simulink model "ofdm_txrx_supermimo_coded"  (Simulink 7.7)
settings: Solver=VariableStepDiscrete  SolverMode=SingleTasking  StartTime=0.0  StopTime=2*simOnly_simLength  RelTol=1e-3
PostLoadFcn:
  | ofdm_rx_supermimo_init
InitFcn:
  | ofdm_rx_supermimo_init
StartFcn:
  | ofdm_rx_supermimo_init
values from neighbouring files (symbols this model uses):
  ActionID_AFTransmit = 31    [ofdm_tx_supermimo_init.m]
  ActionID_Disabled = 0    [ofdm_tx_supermimo_init.m]
  ActionID_SetFlagA = 62    [ofdm_tx_supermimo_init.m]
  ActionID_SetFlagB = 61    [ofdm_tx_supermimo_init.m]
  BER_RAM_init_size = 2048    [ofdm_tx_supermimo_init.m]
  channel_h = [1 0; 1 0] * 0.3    [ofdm_tx_supermimo_init.m]
  CPLength = 16    [ofdm_tx_supermimo_init.m]
  longCorr_coef_i = [-4*real(LTS) 0]    [ofdm_rx_supermimo_init.m]
  longCorr_coef_q = [4*imag(LTS) 0]    [ofdm_rx_supermimo_init.m]
  max_CP_length = 16    [ofdm_tx_supermimo_init.m]
  max_num_baseRateSymbols = 31    [ofdm_tx_supermimo_init.m]
  max_num_subcarriers = 64    [ofdm_tx_supermimo_init.m]
  max_num_trainingSymbols = 15    [ofdm_tx_supermimo_init.m]
  max_numBytes = 16384    [ofdm_tx_supermimo_init.m]
  max_OFDM_symbols = 1023    [ofdm_tx_supermimo_init.m]
  modConstellation_qam16 = .75*[1 3 -1 -3]./3    [ofdm_tx_supermimo_init.m]
  modConstellation_qam64 = 0.875*[3 1 5 7 -3 -1 -5 -7]./7    [ofdm_tx_supermimo_init.m]
  modConstellation_qpsk = [1 -1]./sqrt(2)    [ofdm_tx_supermimo_init.m]
  numBaseRateSymbols = numBaseRateSymbols *2    [ofdm_tx_supermimo_init.m]
  numSubcarriers = 64    [ofdm_tx_supermimo_init.m]
  numTrainingSymbols = 2    [ofdm_tx_supermimo_init.m]
  pktTiming_count_payloadStart = pktTiming_count_lsStart + pktTiming_ls_num*pktTiming_ls_length    [ofdm_rx_supermimo_init.m]
  preamble = preamble_scale*[shortsyms_10 longsyms_2]    [ofdm_tx_supermimo_init.m]
  preamble_ROM = preamble_I_ROM + preamble_Q_ROM*2^16    [ofdm_tx_supermimo_init.m]
  RAM_init_size = max_numBytes    (= 16384)    [ofdm_tx_supermimo_init.m]
  RxDataScrambling_Seq = [40 198 78 63 82 173 102 245 48 111 172 115 147 230 216 93 72 65 62 2 205 242 122 90 128 83 105 97 73 10 5 252]    [ofdm_tx_supermimo_init.m]
  shortSymbol_time = shortSymbol_time(1:16).'    [ofdm_tx_supermimo_init.m]
  simOnly_numSamples = length(preamble)+( (numSubcarriers+CPLength)*(numTrainingSymbols + numBaseRateSymbols + numFullRateSymbols/2) )    [ofdm_tx_supermimo_init.m]
  train = [train train]    [ofdm_tx_supermimo_init.m]
  TxDataScrambling_Seq = [40 198 78 63 82 173 102 245 48 111 172 115 147 230 216 93 72 65 62 2 205 242 122 90 128 83 105 97 73 10 5 252]    [ofdm_tx_supermimo_init.m]
undefined symbols (not defined in the file or in its folder): AntA, AntB, BaseRate, Down, gcbh, numBuffs, numBytes, Up, xlBlockLoadCallback, xlGetNormalizedPeriod, xlipgetpartsetting
libraries: xbsIndex_r4 x5430
(part 13 of 49)

== system ofdm_txrx_supermimo_coded/OFDM Rx MIMO/Long Corr HP/C4 ==
  x : Inport
  AddSub : xbsIndex_r4/AddSub  mode=Addition  use_carryin=off  use_carryout=off  en=off  latency=0  precision=Full  arith_type=Unsigned  n_bits=8  bin_pt=2  quantization=Truncate  overflow=Wrap  dbl_ovrd=off  use_behavioral_HDL=on  hw_selection=Fabric  pipelined=off  use_rpm=off  block_type=addsub
      settings: xl_area=[3 0 0 4 0 0 0]  block_version=VER_STRING_GOES_HERE
  AddSub{1..14} (14 alike) : xbsIndex_r4/AddSub  mode=Addition  use_carryin=off  use_carryout=off  en=off  latency=1  precision=Full  arith_type=Unsigned  n_bits=8  bin_pt=2  quantization=Truncate  overflow=Wrap  dbl_ovrd=off  use_behavioral_HDL=on  hw_selection=Fabric  pipelined=off  use_rpm=off  block_type=addsub
      AddSub1 settings: xl_area=[3 5 0 4 0 0 0]  block_version=VER_STRING_GOES_HERE
      AddSub10 settings: xl_area=[3 5 0 4 0 0 0]  block_version=VER_STRING_GOES_HERE
      AddSub11 settings: xl_area=[3 5 0 4 0 0 0]  block_version=VER_STRING_GOES_HERE
      AddSub12 settings: xl_area=[3 5 0 4 0 0 0]  block_version=VER_STRING_GOES_HERE
      AddSub13 settings: xl_area=[3 6 0 5 0 0 0]  block_version=VER_STRING_GOES_HERE
      AddSub14 settings: xl_area=[3 6 0 5 0 0 0]  block_version=VER_STRING_GOES_HERE
      AddSub2 settings: xl_area=[3 6 0 5 0 0 0]  block_version=VER_STRING_GOES_HERE
      AddSub3 settings: xl_area=[3 5 0 4 0 0 0]  block_version=VER_STRING_GOES_HERE
      AddSub4 settings: xl_area=[4 7 0 6 0 0 0]  block_version=VER_STRING_GOES_HERE
      AddSub5 settings: xl_area=[3 5 0 4 0 0 0]  block_version=VER_STRING_GOES_HERE
      AddSub6 settings: xl_area=[3 6 0 5 0 0 0]  block_version=VER_STRING_GOES_HERE
      AddSub7 settings: xl_area=[3 5 0 4 0 0 0]  block_version=VER_STRING_GOES_HERE
      AddSub8 settings: xl_area=[4 8 0 7 0 0 0]  block_version=VER_STRING_GOES_HERE
      AddSub9 settings: xl_area=[4 7 0 6 0 0 0]  block_version=VER_STRING_GOES_HERE
  C1 : Subsystem  mask{coef2=h(1:N)}
      mask prompts: coef2 "Coefficients"
  C10 : Subsystem  mask{coef2=h(9*N+1:10*N)}
      mask prompts: coef2 "Coefficients"
  C11 : Subsystem  mask{coef2=h(10*N+1:11*N)}
      mask prompts: coef2 "Coefficients"
  C12 : Subsystem  mask{coef2=h(11*N+1:12*N)}
      mask prompts: coef2 "Coefficients"
  C13 : Subsystem  mask{coef2=h(12*N+1:13*N)}
      mask prompts: coef2 "Coefficients"
  C14 : Subsystem  mask{coef2=h(13*N+1:14*N)}
      mask prompts: coef2 "Coefficients"
  C15 : Subsystem  mask{coef2=h(14*N+1:15*N)}
      mask prompts: coef2 "Coefficients"
  C16 : Subsystem  mask{coef2=h(15*N+1:16*N)}
      mask prompts: coef2 "Coefficients"
  C2 : Subsystem  mask{coef2=h(N+1:2*N)}
      mask prompts: coef2 "Coefficients"
  C3 : Subsystem  mask{coef2=h(2*N+1:3*N)}
      mask prompts: coef2 "Coefficients"
  C4 : Subsystem  mask{coef2=h(3*N+1:4*N)}
      mask prompts: coef2 "Coefficients"
  C5 : Subsystem  mask{coef2=h(4*N+1:5*N)}
      mask prompts: coef2 "Coefficients"
  C6 : Subsystem  mask{coef2=h(5*N+1:6*N)}
      mask prompts: coef2 "Coefficients"
  C7 : Subsystem  mask{coef2=h(6*N+1:7*N)}
      mask prompts: coef2 "Coefficients"
  C8 : Subsystem  mask{coef2=h(7*N+1:8*N)}
      mask prompts: coef2 "Coefficients"
  C9 : Subsystem  mask{coef2=h(8*N+1:9*N)}
      mask prompts: coef2 "Coefficients"
  Delay{1,3..4,6,8,12..13,15} (8 alike) : xbsIndex_r4/Delay  rst=off  infoeditControl=Selection of Reset will increase slice count due to use of real FFs and instead of SRLs  en=off  latency=N-1  dbl_ovrd=off  reg_retiming=off  block_type=delay
      settings: infoedit=Hardware notes: A delay line is a chain, each link of which is an SRL16 followed by a flip-flop. If register retiming is enabled, the delay line is a chain of flip-flops.  xl_area=[2 4 0 4 0 0 0]  block_version=VER_STRING_GOES_HERE
  Delay10 : xbsIndex_r4/Delay  rst=off  infoeditControl=Selection of Reset will increase slice count due to use of real FFs and instead of SRLs  en=off  latency=((N-1)*12)-1  dbl_ovrd=off  reg_retiming=off  block_type=delay
      settings: infoedit=Hardware notes: A delay line is a chain, each link of which is an SRL16 followed by a flip-flop. If register retiming is enabled, the delay line is a chain of flip-flops.  xl_area=[9 15 0 15 0 0 0]  block_version=VER_STRING_GOES_HERE
  Delay11 : xbsIndex_r4/Delay  rst=off  infoeditControl=Selection of Reset will increase slice count due to use of real FFs and instead of SRLs  en=off  latency=((N-1)*14)-1  dbl_ovrd=off  reg_retiming=off  block_type=delay
      settings: infoedit=Hardware notes: A delay line is a chain, each link of which is an SRL16 followed by a flip-flop. If register retiming is enabled, the delay line is a chain of flip-flops.  xl_area=[9 15 0 15 0 0 0]  block_version=VER_STRING_GOES_HERE
  Delay14 : xbsIndex_r4/Delay  rst=off  infoeditControl=Selection of Reset will increase slice count due to use of real FFs and instead of SRLs  en=off  latency=((N-1)*2)-1  dbl_ovrd=off  reg_retiming=off  block_type=delay
      settings: infoedit=Hardware notes: A delay line is a chain, each link of which is an SRL16 followed by a flip-flop. If register retiming is enabled, the delay line is a chain of flip-flops.  xl_area=[3 5 0 5 0 0 0]  block_version=VER_STRING_GOES_HERE
  Delay2 : xbsIndex_r4/Delay  rst=off  infoeditControl=Selection of Reset will increase slice count due to use of real FFs and instead of SRLs  en=off  latency=((N-1)*4)-1  dbl_ovrd=off  reg_retiming=off  block_type=delay
      settings: infoedit=Hardware notes: A delay line is a chain, each link of which is an SRL16 followed by a flip-flop. If register retiming is enabled, the delay line is a chain of flip-flops.  xl_area=[3 5 0 5 0 0 0]  block_version=VER_STRING_GOES_HERE
  Delay5 : xbsIndex_r4/Delay  rst=off  infoeditControl=Selection of Reset will increase slice count due to use of real FFs and instead of SRLs  en=off  latency=((N-1)*6)-1  dbl_ovrd=off  reg_retiming=off  block_type=delay
      settings: infoedit=Hardware notes: A delay line is a chain, each link of which is an SRL16 followed by a flip-flop. If register retiming is enabled, the delay line is a chain of flip-flops.  xl_area=[6 10 0 10 0 0 0]  block_version=VER_STRING_GOES_HERE
  Delay7 : xbsIndex_r4/Delay  rst=off  infoeditControl=Selection of Reset will increase slice count due to use of real FFs and instead of SRLs  en=off  latency=((N-1)*8)-1  dbl_ovrd=off  reg_retiming=off  block_type=delay
      settings: infoedit=Hardware notes: A delay line is a chain, each link of which is an SRL16 followed by a flip-flop. If register retiming is enabled, the delay line is a chain of flip-flops.  xl_area=[6 10 0 10 0 0 0]  block_version=VER_STRING_GOES_HERE
  Delay9 : xbsIndex_r4/Delay  rst=off  infoeditControl=Selection of Reset will increase slice count due to use of real FFs and instead of SRLs  en=off  latency=((N-1)*10)-1  dbl_ovrd=off  reg_retiming=off  block_type=delay
      settings: infoedit=Hardware notes: A delay line is a chain, each link of which is an SRL16 followed by a flip-flop. If register retiming is enabled, the delay line is a chain of flip-flops.  xl_area=[9 15 0 15 0 0 0]  block_version=VER_STRING_GOES_HERE
  FSM : Subsystem
  Gateway Out6 : xbsIndex_r4/Gateway Out  inherit_from_input=off  hdl_port=off  timing_constraint=None  locs_specified=off  LOCs={}  UseAsDAC=off  DACChannel='1'  block_type=gatewayout
      settings: infoedit="Gateway out block.  Converts Xilinx fixed point inputs into ouputs of type Simulink integer, double, or fixed point.<P><P>Hardware notes:  In hardware these blocks become top level output ports or are discarded, depending on how they are configured."  xl_area=[0 0 0 0 0 0 0]  block_version=VER_STRING_GOES_HERE
  Gateway Out7 : xbsIndex_r4/Gateway Out  inherit_from_input=off  hdl_port=off  timing_constraint=None  locs_specified=off  LOCs={}  UseAsDAC=off  DACChannel='1'  block_type=gatewayout
      settings: infoedit="Gateway out block.  Converts Xilinx fixed point inputs into ouputs of type Simulink integer, double, or fixed point.<P><P>Hardware notes:  In hardware these blocks become top level output ports or are discarded, depending on how they are configured."  xl_area=[0 0 0 0 0 0 0]  block_version=VER_STRING_GOES_HERE
  Gateway Out8 : xbsIndex_r4/Gateway Out  inherit_from_input=off  hdl_port=off  timing_constraint=None  locs_specified=off  LOCs={}  UseAsDAC=off  DACChannel='1'  block_type=gatewayout
      settings: infoedit="Gateway out block.  Converts Xilinx fixed point inputs into ouputs of type Simulink integer, double, or fixed point.<P><P>Hardware notes:  In hardware these blocks become top level output ports or are discarded, depending on how they are configured."  xl_area=[0 0 0 0 0 0 0]  block_version=VER_STRING_GOES_HERE
  Long Corr Scope2 : Scope  NumInputPorts=3
      settings: YMin=-0.35~-0.175~0  YMax=0.35~0.225~1300  SaveName=ScopeData8
  y : Outport
  wires:
  AddSub9/1 -> Gateway Out8/1, AddSub8/2
  AddSub6/1 -> AddSub9/1, Gateway Out7/1
  C13/1(yn) -> AddSub10/1
  AddSub8/1 -> y/1
  Delay9/1 -> AddSub6/2
  Delay7/1 -> AddSub6/1
  C12/1(yn) -> Delay8/1
  AddSub7/1 -> Delay9/1
  Delay8/1 -> AddSub7/2
  C11/1(yn) -> AddSub7/1
  AddSub4/1 -> AddSub8/1
  AddSub2/1 -> AddSub4/2
  AddSub13/1 -> AddSub4/1
  Delay5/1 -> AddSub2/2
  Delay2/1 -> AddSub2/1
  AddSub/1 -> AddSub13/1
  Delay14/1 -> AddSub13/2
  C8/2(xnz) -> [xnz]
  C10/1(yn) -> Delay6/1
  AddSub5/1 -> Delay7/1
  Delay6/1 -> AddSub5/2
  C9/1(yn) -> AddSub5/1
  C8/1(yn) -> Delay4/1
  C7/1(yn) -> AddSub3/1
  AddSub3/1 -> Delay5/1
  Delay4/1 -> AddSub3/2
  C7/2(xnz) -> C8/1(xn)
  C6/1(yn) -> Delay1/1
  AddSub1/1 -> Delay2/1
  Delay1/1 -> AddSub1/2
  C5/1(yn) -> AddSub1/1
  C1/1(yn) -> AddSub/1
  Delay3/1 -> AddSub/2
  AddSub12/1 -> Delay14/1
  Delay13/1 -> AddSub12/2
  C12/2(xnz) -> C13/1(xn)
  C11/2(xnz) -> C12/1(xn)
  C10/2(xnz) -> C11/1(xn)
  C9/2(xnz) -> C10/1(xn)
  [xnz] -> C9/1(xn)
  C6/2(xnz) -> C7/1(xn)
  C5/2(xnz) -> C6/1(xn)
  C4/1(yn) -> Delay13/1
  C4/2(xnz) -> C5/1(xn)
  C3/1(yn) -> AddSub12/1
  C3/2(xnz) -> C4/1(xn)
  C2/2(xnz) -> C3/1(xn)
  FSM/3(load) -> C12/4(LD), C13/4(LD), C14/4(LD), C16/4(LD), C15/4(LD), C11/4(LD), C10/4(LD), C9/4(LD), C1/4(LD), C8/4(LD), C7/4(LD), C6/4(LD), C5/4(LD), C4/4(LD), C3/4(LD), C2/4(LD)
  FSM/2(Coef Addr) -> C12/3(CAddr), C13/3(CAddr), C14/3(CAddr), C16/3(CAddr), C15/3(CAddr), C11/3(CAddr), C10/3(CAddr), C9/3(CAddr), C1/3(CAddr), C8/3(CAddr), C7/3(CAddr), C6/3(CAddr), C5/3(CAddr), C4/3(CAddr), C3/3(CAddr), C2/3(CAddr)
  FSM/1(Data Addr) -> C12/2(DAddr), C13/2(DAddr), C14/2(DAddr), C16/2(DAddr), C15/2(DAddr), C11/2(DAddr), C10/2(DAddr), C9/2(DAddr), C1/2(DAddr), C8/2(DAddr), C7/2(DAddr), C6/2(DAddr), C5/2(DAddr), C4/2(DAddr), C3/2(DAddr), C2/2(DAddr)
  C1/2(xnz) -> C2/1(xn)
  C2/1(yn) -> Delay3/1
  x/1 -> C1/1(xn)
  C13/2(xnz) -> C14/1(xn)
  C14/2(xnz) -> C15/1(xn)
  C15/2(xnz) -> C16/1(xn)
  C15/1(yn) -> AddSub11/1
  C14/1(yn) -> Delay12/1
  C16/1(yn) -> Delay15/1
  Delay12/1 -> AddSub10/2
  Delay15/1 -> AddSub11/2
  AddSub10/1 -> Delay10/1
  AddSub11/1 -> Delay11/1
  Delay10/1 -> AddSub14/1
  Delay11/1 -> AddSub14/2
  AddSub14/1 -> AddSub9/2, Gateway Out6/1
  Gateway Out6/1 -> Long Corr Scope2/1
  Gateway Out7/1 -> Long Corr Scope2/2
  Gateway Out8/1 -> Long Corr Scope2/3

== system ofdm_txrx_supermimo_coded/OFDM Rx MIMO/Packet_Detection/Auto-Correlation Pkt Det Embedded MULT (for V2P/V6 export) ==
  note: Latency = 1
  note: Latency = 1
  AntA I : Inport
  AntA Q : Inport  Port=2
  PktDet Reset : Inport  Port=3
  A*conj(B) 4xCLK : Subsystem
  Coarse Pkt Det : Scope  NumInputPorts=7
      settings: TimeRange=2500  YMin=-1~-1~0.4~-0.09~12.75~-0.55~4  YMax=1~1~1.3~0.05~13.1~-0.05~4.275  SaveName=ScopeData28
  Coarse Pkt Det2 : Scope  NumInputPorts=7
      settings: TimeRange=2500  YMin=0~0~0~0~0~-1~0.95  YMax=0.3~0.08~0.8~0.7~0.45~1~1.05
  Convert1 : xbsIndex_r4/Convert  gui_display_data_type=Fixed-point  arith_type="Signed  (2's comp)"  n_bits=12  bin_pt=11  float_type=Single  exp_bits=8  fraction_bits=24  quantization=Truncate  overflow=Wrap  en=off  latency=0  dbl_ovrd=off  pipeline=off  block_type=convert
      settings: block_version=10.1.3
  Convert7 : xbsIndex_r4/Convert  gui_display_data_type=Fixed-point  arith_type="Signed  (2's comp)"  n_bits=12  bin_pt=11  float_type=Single  exp_bits=8  fraction_bits=24  quantization=Truncate  overflow=Wrap  en=off  latency=0  dbl_ovrd=off  pipeline=off  block_type=convert
      settings: block_version=10.1.3
  Delay : xbsIndex_r4/Delay  rst=off  infoeditControl=Selection of Reset will increase slice count due to use of real FFs and instead of SRLs  en=off  latency=16  dbl_ovrd=off  reg_retiming=off  block_type=delay
      settings: infoedit=Hardware notes: A delay line is a chain, each link of which is an SRL16 followed by a flip-flop. If register retiming is enabled, the delay line is a chain of flip-flops.  xl_area=[7 14 0 14 0 0 0]  block_version=VER_STRING_GOES_HERE
  Delay1 : xbsIndex_r4/Delay  rst=off  infoeditControl=Selection of Reset will increase slice count due to use of real FFs and instead of SRLs  en=off  latency=16  dbl_ovrd=off  reg_retiming=off  block_type=delay
      settings: infoedit=Hardware notes: A delay line is a chain, each link of which is an SRL16 followed by a flip-flop. If register retiming is enabled, the delay line is a chain of flip-flops.  xl_area=[7 14 0 14 0 0 0]  block_version=VER_STRING_GOES_HERE
  Detection Decision : Subsystem
  Display{,1..3} (4 alike) : Display
  Logical : xbsIndex_r4/Logical  logical_function=AND  inputs=2  en=off  latency=0  precision=Full  arith_type=Unsigned  n_bits=8  bin_pt=2  align_bp=on  dbl_ovrd=off  block_type=logical
      settings: xl_area=[1 0 0 1 0 0 0]  block_version=VER_STRING_GOES_HERE
  Magnitude Squared : Subsystem
  Magnitude Squared1 : Subsystem
  Mult1 : xbsIndex_r4/Mult  precision=User Defined  arith_type=Unsigned  n_bits=30  bin_pt=20  quantization=Truncate  overflow=Flag as error  en=off  latency=1  dbl_ovrd=off  use_behavioral_HDL=off  opt=Speed  use_embedded=on  optimum_pipeline=off  pipeline=on  use_rpm=off  placement_style=Rectangular shape  block_type=mult
      settings: xl_area=[63 105 0 106 0 4 0]  block_version=VER_STRING_GOES_HERE
  Mult2 : xbsIndex_r4/Mult  precision=User Defined  arith_type=Unsigned  n_bits=30  bin_pt=20  quantization=Truncate  overflow=Saturate  en=off  latency=1  dbl_ovrd=off  use_behavioral_HDL=off  opt=Speed  use_embedded=on  optimum_pipeline=off  pipeline=on  use_rpm=off  placement_style=Rectangular shape  block_type=mult
      settings: xl_area=[63 105 0 106 0 4 0]  block_version=VER_STRING_GOES_HERE
  Reinterpret : xbsIndex_r4/Reinterpret  force_arith_type=on  arith_type=Unsigned  force_bin_pt=on  bin_pt=0  block_type=reinterpret
  Reinterpret1 : xbsIndex_r4/Reinterpret  force_arith_type=on  arith_type=Unsigned  force_bin_pt=on  bin_pt=0  block_type=reinterpret
  Relational : xbsIndex_r4/Relational  mode=a>b  en=off  latency=1  dbl_ovrd=off  block_type=relational
      settings: xl_area=[11 1 0 22 0 0 0]  block_version=VER_STRING_GOES_HERE
  Relational1 : xbsIndex_r4/Relational  mode=a>b  en=off  latency=1  dbl_ovrd=off  block_type=relational
      settings: xl_area=[11 1 0 22 0 0 0]  block_version=VER_STRING_GOES_HERE
  Sliding Window : Subsystem
  Sliding Window1 : Subsystem
  Sliding Window2 : Subsystem
  done{1..18} (18 alike) : xbsIndex_r4/Gateway Out  inherit_from_input=off  hdl_port=off  timing_constraint=None  locs_specified=off  LOCs={}  UseAsDAC=off  DACChannel='1'  block_type=gatewayout
      settings: infoedit="Gateway out block.  Converts Xilinx fixed point inputs into ouputs of type Simulink integer, double, or fixed point.<P><P>Hardware notes:  In hardware these blocks become top level output ports or are discarded, depending on how they are configured."  xl_area=[0 0 0 0 1 0 0]  block_version=VER_STRING_GOES_HERE
  PktDet : Outport
  wires:
  Logical/1 -> Detection Decision/1(Det)
  Sliding Window2/1(Out) -> done10/1, Mult2/2, Mult2/1
  [pktDetCorr_minPower] -> Relational1/2, done15/1, Reinterpret1/1
  done13/1 -> Coarse Pkt Det2/6
  done12/1 -> Coarse Pkt Det2/5
  Mult2/1 -> Mult1/1, done11/1, Relational1/1
  [pktDetCorr_ratioThresh] -> Mult1/2, done14/1, Reinterpret/1
  done11/1 -> Coarse Pkt Det2/4
  done10/1 -> Coarse Pkt Det2/3
  done9/1 -> Coarse Pkt Det2/2
  done8/1 -> Coarse Pkt Det2/1
  Mult1/1 -> done12/1, Relational/2
  Magnitude Squared1/1(Mag) -> done9/1, Sliding Window2/1(In)
  done7/1 -> Coarse Pkt Det/7
  done3/1 -> Coarse Pkt Det/4
  Convert1/1 -> Magnitude Squared1/2(Q), done2/1, Delay1/1, A*conj(B) 4xCLK/3(Im[A])
  Convert7/1 -> Magnitude Squared1/1(I), done4/1, Delay/1, A*conj(B) 4xCLK/1(Re[A])
  done2/1 -> Coarse Pkt Det/2
  AntA Q/1 -> Convert1/1
  AntA I/1 -> Convert7/1
  Delay/1 -> A*conj(B) 4xCLK/2(Re[B])
  Delay1/1 -> A*conj(B) 4xCLK/4(Im[B])
  A*conj(B) 4xCLK/1(Re) -> done5/1, Sliding Window/1(In)
  A*conj(B) 4xCLK/2(Im1) -> done3/1, Sliding Window1/1(In)
  Sliding Window/1(Out) -> done6/1, Magnitude Squared/1(I)
  Sliding Window1/1(Out) -> done1/1, Magnitude Squared/2(Q)
  Magnitude Squared/1(Mag) -> Relational/1, done8/1, done7/1
  Relational/1 -> done13/1, Logical/1
  Relational1/1 -> Logical/2, done16/1
  done1/1 -> Coarse Pkt Det/6
  done4/1 -> Coarse Pkt Det/1
  done5/1 -> Coarse Pkt Det/3
  done6/1 -> Coarse Pkt Det/5
  done14/1 -> Display/1
  done15/1 -> Display1/1
  done16/1 -> Coarse Pkt Det2/7
  Reinterpret/1 -> done17/1
  done17/1 -> Display2/1
  Reinterpret1/1 -> done18/1
  done18/1 -> Display3/1
  PktDet Reset/1 -> Detection Decision/2(Reset)
  Detection Decision/1(Pkt Det) -> PktDet/1

== system ofdm_txrx_supermimo_coded/OFDM Rx MIMO/Packet_Detection/Ext PktDet ==
  Constant2 : Constant  Value=0
  Convert5 : xbsIndex_r4/Convert  gui_display_data_type=Fixed-point  arith_type=Boolean  n_bits=1  bin_pt=0  float_type=Single  exp_bits=8  fraction_bits=24  quantization=Truncate  overflow=Flag as error  en=off  latency=0  dbl_ovrd=off  pipeline=off  block_type=convert
      settings: block_version=10.1.3
  Down Sample1 : xbsIndex_r4/Down Sample  sample_ratio=4  sample_phase="Last Value of Frame  (most efficient)"  en=off  latency=1  dbl_ovrd=off  block_type=dsamp
      settings: block_version=VER_STRING_GOES_HERE
  Ext_PktDet : xbsIndex_r4/Gateway In  gui_display_data_type=Fixed-point  arith_type=Unsigned  n_bits=1  bin_pt=0  preci_type=Single  exp_width=8  frac_width=24  quantization="Round  (unbiased: +/- Inf)"  overflow=Saturate  period=1  dbl_ovrd=off  timing_constraint=None  locs_specified=off  LOCs={}  inherit_from_input=off  UseAsADC=off  ADCChannel='1'  hdl_port=on  block_type=gatewayin
      settings: infoedit="Gateway in block.  Converts inputs of type Simulink integer, double and fixed point to  Xilinx fixed point type.<P><P>Hardware notes:  In hardware these blocks become top level input ports."  block_version=VER_STRING_GOES_HERE
  Logical2 : xbsIndex_r4/Logical  logical_function=AND  inputs=2  en=off  latency=0  precision=Full  arith_type=Unsigned  n_bits=16  bin_pt=0  align_bp=on  dbl_ovrd=off  block_type=logical
      settings: block_version=8.2
  Det : Outport
  wires:
  Ext_PktDet/1 -> Down Sample1/1
  Constant2/1 -> Ext_PktDet/1
  [regPktDet_EnableExtDet] -> Logical2/2
  Logical2/1 -> Det/1
  Convert5/1 -> Logical2/1
  Down Sample1/1 -> Convert5/1

== system ofdm_txrx_supermimo_coded/OFDM Rx MIMO/Packet_Detection/Posedge ==
  D : Inport
  Delay : xbsIndex_r4/Delay  rst=off  infoeditControl=Selection of Reset will increase slice count due to use of real FFs and instead of SRLs  en=off  latency=1  dbl_ovrd=off  reg_retiming=off  block_type=delay
      settings: infoedit=Hardware notes: A delay line is a chain, each link of which is an SRL16 followed by a flip-flop. If register retiming is enabled, the delay line is a chain of flip-flops.  xl_area=[1 1 0 0 0 0 0]  block_version=VER_STRING_GOES_HERE
  Inverter : xbsIndex_r4/Inverter  en=off  latency=0  dbl_ovrd=off  block_type=inv
      settings: xl_area=[0 0 0 0 0 0 0]  block_version=VER_STRING_GOES_HERE
  Logical : xbsIndex_r4/Logical  logical_function=AND  inputs=2  en=off  latency=0  precision=Full  arith_type=Unsigned  n_bits=8  bin_pt=2  align_bp=on  dbl_ovrd=off  block_type=logical
      settings: xl_area=[1 0 0 1 0 0 0]  block_version=VER_STRING_GOES_HERE
  Q : Outport
  wires:
  Logical/1 -> Q/1
  D/1 -> Delay/1, Logical/1
  Inverter/1 -> Logical/2
  Delay/1 -> Inverter/1

== system ofdm_txrx_supermimo_coded/OFDM Rx MIMO/Packet_Detection/RSSI-based PktDet ==
  Payload : Inport
  Force Busy : Inport  Port=2
  PktDet Reset : Inport  Port=3
  Ant A Carrier Sensing : Subsystem
  Ant B Carrier Sensing : Subsystem
  AntA Detector : Subsystem
  AntB Detector : Subsystem
  Constant1 : Constant  Value=100
  Constant2 : Constant  Value=0
  Constant3 : Constant  Value=0
  Convert3 : xbsIndex_r4/Convert  gui_display_data_type=Fixed-point  arith_type=Unsigned  n_bits=1  bin_pt=0  float_type=Single  exp_bits=8  fraction_bits=24  quantization=Truncate  overflow=Wrap  en=off  latency=0  dbl_ovrd=off  pipeline=off  block_type=convert
      settings: block_version=10.1.3
  Convert5 : xbsIndex_r4/Convert  gui_display_data_type=Fixed-point  arith_type=Boolean  n_bits=1  bin_pt=0  float_type=Single  exp_bits=8  fraction_bits=24  quantization=Truncate  overflow=Flag as error  en=off  latency=0  dbl_ovrd=off  pipeline=off  block_type=convert
      settings: block_version=10.1.3
  Convert7 : xbsIndex_r4/Convert  gui_display_data_type=Fixed-point  arith_type=Unsigned  n_bits=1  bin_pt=0  float_type=Single  exp_bits=8  fraction_bits=24  quantization=Truncate  overflow=Wrap  en=off  latency=0  dbl_ovrd=off  pipeline=off  block_type=convert
      settings: block_version=10.1.3
  DIFS Counter : Subsystem
  Down Sample1 : xbsIndex_r4/Down Sample  sample_ratio=4  sample_phase="Last Value of Frame  (most efficient)"  en=off  latency=1  dbl_ovrd=off  block_type=dsamp
      settings: block_version=VER_STRING_GOES_HERE
  Down Sample2 : xbsIndex_r4/Down Sample  sample_ratio=4  sample_phase="Last Value of Frame  (most efficient)"  en=off  latency=1  dbl_ovrd=off  block_type=dsamp
      settings: block_version=VER_STRING_GOES_HERE
  Down Sample3 : xbsIndex_r4/Down Sample  sample_ratio=4  sample_phase="Last Value of Frame  (most efficient)"  en=off  latency=1  dbl_ovrd=off  block_type=dsamp
      settings: block_version=VER_STRING_GOES_HERE
  Gateway Out : xbsIndex_r4/Gateway Out  inherit_from_input=off  hdl_port=off  timing_constraint=None  locs_specified=off  LOCs={}  UseAsDAC=off  DACChannel='1'  block_type=gatewayout
      settings: infoedit="Gateway out block.  Converts Xilinx fixed point inputs into ouputs of type Simulink integer, double, or fixed point.<P><P>Hardware notes:  In hardware these blocks become top level output ports or are discarded, depending on how they are configured."  block_version=8.2
  Gateway Out1 : xbsIndex_r4/Gateway Out  inherit_from_input=off  hdl_port=off  timing_constraint=None  locs_specified=off  LOCs={}  UseAsDAC=off  DACChannel='1'  block_type=gatewayout
      settings: infoedit="Gateway out block.  Converts Xilinx fixed point inputs into ouputs of type Simulink integer, double, or fixed point.<P><P>Hardware notes:  In hardware these blocks become top level output ports or are discarded, depending on how they are configured."  block_version=8.2
  Gateway Out2 : xbsIndex_r4/Gateway Out  inherit_from_input=off  hdl_port=off  timing_constraint=None  locs_specified=off  LOCs={}  UseAsDAC=off  DACChannel='1'  block_type=gatewayout
      settings: infoedit="Gateway out block.  Converts Xilinx fixed point inputs into ouputs of type Simulink integer, double, or fixed point.<P><P>Hardware notes:  In hardware these blocks become top level output ports or are discarded, depending on how they are configured."  block_version=8.2
  IDLEFORDIFS : xbsIndex_r4/Gateway Out  inherit_from_input=off  hdl_port=on  timing_constraint=None  locs_specified=off  LOCs={}  UseAsDAC=off  DACChannel='1'  block_type=gatewayout
      settings: infoedit="Gateway out block.  Converts Xilinx fixed point inputs into ouputs of type Simulink integer, double, or fixed point.<P><P>Hardware notes:  In hardware these blocks become top level output ports or are discarded, depending on how they are configured."  block_version=VER_STRING_GOES_HERE
  IDLEFORDIFS_disable : xbsIndex_r4/Gateway In  gui_display_data_type=Fixed-point  arith_type=Unsigned  n_bits=1  bin_pt=0  preci_type=Single  exp_width=8  frac_width=24  quantization="Round  (unbiased: +/- Inf)"  overflow=Saturate  period=1  dbl_ovrd=off  timing_constraint=None  locs_specified=off  LOCs={}  inherit_from_input=off  UseAsADC=off  ADCChannel='1'  hdl_port=on  block_type=gatewayin
      settings: infoedit="Gateway in block.  Converts inputs of type Simulink integer, double and fixed point to  Xilinx fixed point type.<P><P>Hardware notes:  In hardware these blocks become top level input ports."  block_version=VER_STRING_GOES_HERE
  Inverter1 : xbsIndex_r4/Inverter  en=off  latency=0  dbl_ovrd=off  block_type=inv
      settings: block_version=8.2
  Inverter9 : xbsIndex_r4/Inverter  en=off  latency=0  dbl_ovrd=off  block_type=inv
      settings: block_version=8.2
  Logical11 : xbsIndex_r4/Logical  logical_function=AND  inputs=3  en=off  latency=0  precision=Full  arith_type=Unsigned  n_bits=16  bin_pt=0  align_bp=on  dbl_ovrd=off  block_type=logical
      settings: block_version=8.2
  Logical13 : xbsIndex_r4/Logical  logical_function=AND  inputs=3  en=off  latency=0  precision=Full  arith_type=Unsigned  n_bits=16  bin_pt=0  align_bp=on  dbl_ovrd=off  block_type=logical
      settings: block_version=8.2
  Logical5 : xbsIndex_r4/Logical  logical_function=OR  inputs=3  en=off  latency=0  precision=Full  arith_type=Unsigned  n_bits=16  bin_pt=0  align_bp=on  dbl_ovrd=off  block_type=logical
      settings: block_version=8.2
  MIMO Logic : Subsystem
  Mid-Packet RSSI Register : Subsystem
  Pkt Det Decision : Scope  NumInputPorts=3
      settings: YMin=-1~-1~-1  YMax=1~1~1  SaveName=ScopeData54
  RSSI Clock Generator : Subsystem
  RSSI_AntA : xbsIndex_r4/Gateway In  gui_display_data_type=Fixed-point  arith_type=Unsigned  n_bits=10  bin_pt=0  preci_type=Single  exp_width=8  frac_width=24  quantization="Round  (unbiased: +/- Inf)"  overflow=Saturate  period=1  dbl_ovrd=off  timing_constraint=None  locs_specified=off  LOCs={}  inherit_from_input=off  UseAsADC=off  ADCChannel='1'  hdl_port=on  block_type=gatewayin
      settings: infoedit="Gateway in block.  Converts inputs of type Simulink integer, double and fixed point to  Xilinx fixed point type.<P><P>Hardware notes:  In hardware these blocks become top level input ports."  block_version=VER_STRING_GOES_HERE
  RSSI_AntB : xbsIndex_r4/Gateway In  gui_display_data_type=Fixed-point  arith_type=Unsigned  n_bits=10  bin_pt=0  preci_type=Single  exp_width=8  frac_width=24  quantization="Round  (unbiased: +/- Inf)"  overflow=Saturate  period=1  dbl_ovrd=off  timing_constraint=None  locs_specified=off  LOCs={}  inherit_from_input=off  UseAsADC=off  ADCChannel='1'  hdl_port=on  block_type=gatewayin
      settings: infoedit="Gateway in block.  Converts inputs of type Simulink integer, double and fixed point to  Xilinx fixed point type.<P><P>Hardware notes:  In hardware these blocks become top level input ports."  block_version=VER_STRING_GOES_HERE
  Up Sample7 : xbsIndex_r4/Up Sample  sample_ratio=4  copy_samples=on  en=off  latency=0  dbl_ovrd=off  block_type=usamp
      settings: infoedit="Up samples input data.  Inserted values  can be zeros or copies of the most recent input sample.<P><P>Hardware notes: No hardware is needed if inserted values are copies of the input sample; otherwise, a mux and single bit flip-flop are used."  block_version=8.2
  PktDet : Outport
  tag scope: regPktDet_idleForDIFS=global
  wires:
  Constant1/1 -> RSSI_AntA/1
  RSSI_AntA/1 -> Down Sample1/1
  Constant3/1 -> RSSI_AntB/1
  RSSI_AntB/1 -> Down Sample2/1
  Down Sample1/1 -> AntA Detector/1(RSSI)
  Down Sample2/1 -> AntB Detector/1(RSSI)
  AntA Detector/1(pktdet) -> MIMO Logic/1(Det A)
  AntB Detector/1(pktdet) -> MIMO Logic/2(Det B)
  MIMO Logic/1(PktDet) -> Convert5/1
  AntA Detector/2(avgRSSI) -> Ant A Carrier Sensing/1(Avg RSSI), Mid-Packet RSSI Register/2(AntA)
  AntB Detector/2(avgRSSI) -> Ant B Carrier Sensing/1(Avg RSSI), Mid-Packet RSSI Register/1(AntB)
  Ant A Carrier Sensing/1(busy) -> Logical5/1
  Ant B Carrier Sensing/1(busy) -> Logical5/2
  Ant A Carrier Sensing/2(idle) -> Logical11/1, Gateway Out1/1
  Ant B Carrier Sensing/2(idle) -> Logical11/2
  Inverter9/1 -> Logical11/3
  Force Busy/1 -> Convert7/1
  [regPktDet_CSMAenableIdle] -> Convert3/1
  Logical13/1 -> Up Sample7/1
  Up Sample7/1 -> IDLEFORDIFS/1, [regPktDet_idleForDIFS]
  PktDet Reset/1 -> AntA Detector/2(Reset), AntB Detector/2(Reset)
  Convert7/1 -> Logical5/3, Inverter9/1
  Convert3/1 -> Logical13/2
  Payload/1 -> Mid-Packet RSSI Register/3(Payload)
  Logical11/1 -> DIFS Counter/2(Idle)
  Logical5/1 -> DIFS Counter/1(Busy)
  DIFS Counter/1(Idle for DIFS) -> Logical13/1, Gateway Out2/1
  Gateway Out/1 -> Pkt Det Decision/1
  Gateway Out1/1 -> Pkt Det Decision/2
  Gateway Out2/1 -> Pkt Det Decision/3
  Constant2/1 -> IDLEFORDIFS_disable/1
  IDLEFORDIFS_disable/1 -> Down Sample3/1
  Down Sample3/1 -> Inverter1/1
  Inverter1/1 -> Logical13/3
  Convert5/1 -> PktDet/1, Gateway Out/1

== system ofdm_txrx_supermimo_coded/OFDM Rx MIMO/Packet_Timing_Control/Copy_Downsample ==
  D : Inport
  Delay : xbsIndex_r4/Delay  rst=off  infoeditControl=Selection of Reset will increase slice count due to use of real FFs and instead of SRLs  en=off  latency=1  dbl_ovrd=off  reg_retiming=off  block_type=delay
      settings: infoedit=Hardware notes: A delay line is a chain, each link of which is an SRL16 followed by a flip-flop. If register retiming is enabled, the delay line is a chain of flip-flops.  block_version=VER_STRING_GOES_HERE
  Down Sample : xbsIndex_r4/Down Sample  sample_ratio=2  sample_phase="Last Value of Frame  (most efficient)"  en=off  latency=1  dbl_ovrd=off  block_type=dsamp
      settings: block_version=VER_STRING_GOES_HERE
  Logical : xbsIndex_r4/Logical  logical_function=OR  inputs=2  en=off  latency=0  precision=Full  arith_type=Unsigned  n_bits=16  bin_pt=0  align_bp=on  dbl_ovrd=off  block_type=logical
      settings: block_version=VER_STRING_GOES_HERE
  Q : Outport
  wires:
  Down Sample/1 -> Q/1
  Logical/1 -> Down Sample/1
  D/1 -> Logical/2, Delay/1
  Delay/1 -> Logical/1

== system ofdm_txrx_supermimo_coded/OFDM Rx MIMO/Packet_Timing_Control/Disable for Interrupts ==
  PktDone : Inport
  Convert : xbsIndex_r4/Convert  gui_display_data_type=Fixed-point  arith_type=Boolean  n_bits=16  bin_pt=14  float_type=Single  exp_bits=8  fraction_bits=24  quantization=Truncate  overflow=Wrap  en=off  latency=0  dbl_ovrd=off  pipeline=off  block_type=convert
      settings: block_version=8.2.01
  Convert1 : xbsIndex_r4/Convert  gui_display_data_type=Fixed-point  arith_type=Boolean  n_bits=16  bin_pt=14  float_type=Single  exp_bits=8  fraction_bits=24  quantization=Truncate  overflow=Wrap  en=off  latency=0  dbl_ovrd=off  pipeline=off  block_type=convert
      settings: block_version=8.2.01
  Down Sample1 : xbsIndex_r4/Down Sample  sample_ratio=2  sample_phase="Last Value of Frame  (most efficient)"  en=off  latency=1  dbl_ovrd=off  block_type=dsamp
      settings: block_version=8.2.01
  Logical1 : xbsIndex_r4/Logical  logical_function=NOR  inputs=4  en=off  latency=0  precision=Full  arith_type=Unsigned  n_bits=16  bin_pt=0  align_bp=on  dbl_ovrd=off  block_type=logical
      settings: block_version=VER_STRING_GOES_HERE
  Logical2 : xbsIndex_r4/Logical  logical_function=AND  inputs=2  en=off  latency=0  precision=Full  arith_type=Unsigned  n_bits=16  bin_pt=0  align_bp=on  dbl_ovrd=off  block_type=logical
      settings: block_version=VER_STRING_GOES_HERE
  Logical4 : xbsIndex_r4/Logical  logical_function=OR  inputs=2  en=off  latency=0  precision=Full  arith_type=Unsigned  n_bits=16  bin_pt=0  align_bp=on  dbl_ovrd=off  block_type=logical
      settings: block_version=VER_STRING_GOES_HERE
  Logical5 : xbsIndex_r4/Logical  logical_function=OR  inputs=4  en=off  latency=0  precision=Full  arith_type=Unsigned  n_bits=16  bin_pt=0  align_bp=on  dbl_ovrd=off  block_type=logical
      settings: block_version=VER_STRING_GOES_HERE
  Rx PktDet Disable : Scope  NumInputPorts=8
      settings: TimeRange=10000  YMin=0~-1~0~-1~0~0~0~0  YMax=1~1~1~1~1~1~1~1  SaveName=ScopeData34
  S-R Latch : Subsystem
  Up Sample : xbsIndex_r4/Up Sample  sample_ratio=2  copy_samples=on  en=off  latency=0  dbl_ovrd=off  block_type=usamp
      settings: infoedit="Up samples input data.  Inserted values  can be zeros or copies of the most recent input sample.<P><P>Hardware notes: No hardware is needed if inserted values are copies of the input sample; otherwise, a mux and single bit flip-flop are used."  block_version=10.1.3
  done{1..8} (8 alike) : xbsIndex_r4/Gateway Out  inherit_from_input=off  hdl_port=off  timing_constraint=None  locs_specified=off  LOCs={}  UseAsDAC=off  DACChannel='1'  block_type=gatewayout
      settings: infoedit="Gateway out block.  Converts Xilinx fixed point inputs into ouputs of type Simulink integer, double, or fixed point.<P><P>Hardware notes:  In hardware these blocks become top level output ports or are discarded, depending on how they are configured."  xl_area=[0 0 0 0 1 0 0]  block_version=VER_STRING_GOES_HERE
  Det Disabled : Outport
  wires:
  Convert/1 -> Logical2/2
  Logical5/1 -> Convert/1
  [intOut_GoodPkt] -> Logical5/1, Logical1/1, done1/1
  [intOut_BadPkt] -> Logical5/2, Logical1/2, done2/1
  [intOut_GoodHeader] -> Logical5/3, Logical1/3, done3/1
  [intOut_BadHeader] -> Logical5/4, Logical1/4, done4/1
  S-R Latch/1(Q) -> done8/1, Down Sample1/1
  Logical2/1 -> done6/1, S-R Latch/1(S)
  PktDone/1 -> Up Sample/1
  Logical4/1 -> S-R Latch/2(R), done7/1
  [rx_global_reset] -> Logical4/2
  Logical1/1 -> Convert1/1
  Convert1/1 -> Logical4/1
  done4/1 -> Rx PktDet Disable/4
  done3/1 -> Rx PktDet Disable/3
  done2/1 -> Rx PktDet Disable/2
  done1/1 -> Rx PktDet Disable/1
  done5/1 -> Rx PktDet Disable/5
  done6/1 -> Rx PktDet Disable/6
  done7/1 -> Rx PktDet Disable/7
  done8/1 -> Rx PktDet Disable/8
  Down Sample1/1 -> Det Disabled/1
  Up Sample/1 -> Logical2/1, done5/1

== system ofdm_txrx_supermimo_coded/OFDM Rx MIMO/Packet_Timing_Control/Edge Det ==
  note: Rising Edge Detection
  note: Debounce
  D : Inport
  Delay : xbsIndex_r4/Delay  rst=off  infoeditControl=Selection of Reset will increase slice count due to use of real FFs and instead of SRLs  en=off  latency=1  dbl_ovrd=off  reg_retiming=off  block_type=delay
      settings: infoedit=Hardware notes: A delay line is a chain, each link of which is an SRL16 followed by a flip-flop. If register retiming is enabled, the delay line is a chain of flip-flops.  xl_area=[1 1 0 0 0 0 0]  block_version=VER_STRING_GOES_HERE
  Inverter : xbsIndex_r4/Inverter  en=off  latency=0  dbl_ovrd=off  block_type=inv
      settings: xl_area=[0 0 0 0 0 0 0]  block_version=VER_STRING_GOES_HERE
  Logical1 : xbsIndex_r4/Logical  logical_function=AND  inputs=2  en=off  latency=0  precision=Full  arith_type=Unsigned  n_bits=8  bin_pt=2  align_bp=on  dbl_ovrd=off  block_type=logical
      settings: xl_area=[1 0 0 1 0 0 0]  block_version=VER_STRING_GOES_HERE
  Q : Outport
  wires:
  D/1 -> Logical1/2, Delay/1
  Logical1/1 -> Q/1
  Inverter/1 -> Logical1/1
  Delay/1 -> Inverter/1

== system ofdm_txrx_supermimo_coded/OFDM Rx MIMO/Packet_Timing_Control/Edge Det1 ==
  note: Rising Edge Detection
  D : Inport
  Delay : xbsIndex_r4/Delay  rst=off  infoeditControl=Selection of Reset will increase slice count due to use of real FFs and instead of SRLs  en=off  latency=1  dbl_ovrd=off  reg_retiming=off  block_type=delay
      settings: infoedit=Hardware notes: A delay line is a chain, each link of which is an SRL16 followed by a flip-flop. If register retiming is enabled, the delay line is a chain of flip-flops.  xl_area=[1 1 0 0 0 0 0]  block_version=VER_STRING_GOES_HERE
  Inverter : xbsIndex_r4/Inverter  en=off  latency=0  dbl_ovrd=off  block_type=inv
      settings: xl_area=[0 0 0 0 0 0 0]  block_version=VER_STRING_GOES_HERE
  Logical1 : xbsIndex_r4/Logical  logical_function=AND  inputs=2  en=off  latency=0  precision=Full  arith_type=Unsigned  n_bits=8  bin_pt=2  align_bp=on  dbl_ovrd=off  block_type=logical
      settings: xl_area=[1 0 0 1 0 0 0]  block_version=VER_STRING_GOES_HERE
  Q : Outport
  wires:
  Delay/1 -> Inverter/1
  Inverter/1 -> Logical1/1
  Logical1/1 -> Q/1
  D/1 -> Delay/1, Logical1/2

== system ofdm_txrx_supermimo_coded/OFDM Rx MIMO/Packet_Timing_Control/Ext Reset ==
  Convert2 : xbsIndex_r4/Convert  gui_display_data_type=Fixed-point  arith_type=Boolean  n_bits=1  bin_pt=0  float_type=Single  exp_bits=8  fraction_bits=24  quantization=Truncate  overflow=Wrap  en=off  latency=0  dbl_ovrd=off  pipeline=off  block_type=convert
      settings: xl_area=[0, 0, 0, 0, 0, 0, 0]  block_version=VER_STRING_GOES_HERE
  Down Sample : xbsIndex_r4/Down Sample  sample_ratio=4  sample_phase="Last Value of Frame  (most efficient)"  en=off  latency=1  dbl_ovrd=off  block_type=dsamp
      settings: block_version=VER_STRING_GOES_HERE
  Logical10 : xbsIndex_r4/Logical  logical_function=AND  inputs=2  en=off  latency=0  precision=Full  arith_type=Unsigned  n_bits=8  bin_pt=2  align_bp=on  dbl_ovrd=off  block_type=logical
      settings: xl_area=[1 0 0 1 0 0 0]  block_version=VER_STRING_GOES_HERE
  RXreset : Constant  Value=0
  Rx_PktDetReset_In : xbsIndex_r4/Gateway In  gui_display_data_type=Fixed-point  arith_type=Unsigned  n_bits=1  bin_pt=0  preci_type=Single  exp_width=8  frac_width=24  quantization="Round  (unbiased: +/- Inf)"  overflow=Saturate  period=1  dbl_ovrd=off  timing_constraint=None  locs_specified=off  LOCs={}  inherit_from_input=off  UseAsADC=off  ADCChannel='1'  hdl_port=on  block_type=gatewayin
      settings: infoedit="Gateway in block.  Converts inputs of type Simulink integer, double and fixed point to  Xilinx fixed point type.<P><P>Hardware notes:  In hardware these blocks become top level input ports."  block_version=VER_STRING_GOES_HERE
  debounce : Subsystem
  Rst : Outport
  wires:
  [regRx_ExtPktDetReset_En] -> Logical10/1
  Down Sample/1 -> Logical10/2
  RXreset/1 -> Rx_PktDetReset_In/1
  Rx_PktDetReset_In/1 -> Convert2/1
  Convert2/1 -> Down Sample/1
  Logical10/1 -> debounce/1(D)
  debounce/1(Q) -> Rst/1

== system ofdm_txrx_supermimo_coded/OFDM Rx MIMO/Packet_Timing_Control/Fine_Pkt_det ==
  Samp Count : Inport
  Long CorrA : Inport  Port=2
  Delay : xbsIndex_r4/Delay  rst=off  infoeditControl=Selection of Reset will increase slice count due to use of real FFs and instead of SRLs  en=off  latency=64  dbl_ovrd=off  reg_retiming=off  block_type=delay
      settings: infoedit=Hardware notes: A delay line is a chain, each link of which is an SRL16 followed by a flip-flop. If register retiming is enabled, the delay line is a chain of flip-flops.  block_version=VER_STRING_GOES_HERE
  Logical1 : xbsIndex_r4/Logical  logical_function=AND  inputs=2  en=off  latency=0  precision=Full  arith_type=Unsigned  n_bits=8  bin_pt=2  align_bp=on  dbl_ovrd=off  block_type=logical
      settings: xl_area=[1 0 0 1 0 0 0]  block_version=VER_STRING_GOES_HERE
  Logical2 : xbsIndex_r4/Logical  logical_function=AND  inputs=3  en=off  latency=0  precision=Full  arith_type=Unsigned  n_bits=8  bin_pt=2  align_bp=on  dbl_ovrd=off  block_type=logical
      settings: xl_area=[1 0 0 1 0 0 0]  block_version=VER_STRING_GOES_HERE
  Long Corr Pkt Det : Scope  NumInputPorts=5
      settings: TimeRange=2500  YMin=0~-1~0~0~0  YMax=37500~1~1~1~350  SaveName=ScopeData10
  Relational1 : xbsIndex_r4/Relational  mode=a>b  en=off  latency=0  dbl_ovrd=off  block_type=relational
      settings: xl_area=[10 0 0 19 0 0 0]  block_version=VER_STRING_GOES_HERE
  Relational2 : xbsIndex_r4/Relational  mode=a>b  en=off  latency=0  dbl_ovrd=off  block_type=relational
      settings: xl_area=[10 0 0 19 0 0 0]  block_version=VER_STRING_GOES_HERE
  Relational3 : xbsIndex_r4/Relational  mode=a<=b  en=off  latency=0  dbl_ovrd=off  block_type=relational
      settings: xl_area=[10 0 0 19 0 0 0]  block_version=VER_STRING_GOES_HERE
  done{1,3..5} (4 alike) : xbsIndex_r4/Gateway Out  inherit_from_input=off  hdl_port=off  timing_constraint=None  locs_specified=off  LOCs={}  UseAsDAC=off  DACChannel='1'  block_type=gatewayout
      settings: infoedit="Gateway out block.  Converts Xilinx fixed point inputs into ouputs of type Simulink integer, double, or fixed point.<P><P>Hardware notes:  In hardware these blocks become top level output ports or are discarded, depending on how they are configured."  xl_area=[0 0 0 0 1 0 0]  block_version=VER_STRING_GOES_HERE
  Det : Outport
  tag scope: longCorrAboveThresh_Twice=global
  wires:
  Delay/1 -> Logical1/2
  Logical2/1 -> Delay/1, Logical1/1
  Samp Count/1 -> Relational1/1, Relational3/1, done5/1
  done4/1 -> Long Corr Pkt Det/4
  done3/1 -> Long Corr Pkt Det/3
  ? -> Long Corr Pkt Det/2
  done1/1 -> Long Corr Pkt Det/1
  [regRx_PktDet_LongCorr_WindStart] -> Relational1/2
  Relational1/1 -> Logical2/2
  [regRx_PktDet_LongCorr_WindEnd] -> Relational3/2
  Relational3/1 -> Logical2/3
  [regRx_PktDet_LongCorr_Thresh] -> Relational2/2
  Long CorrA/1 -> Relational2/1, done1/1
  Relational2/1 -> Logical2/1, done3/1
  Logical1/1 -> Det/1, [longCorrAboveThresh_Twice], done4/1
  done5/1 -> Long Corr Pkt Det/5

== system ofdm_txrx_supermimo_coded/OFDM Rx MIMO/Packet_Timing_Control/S-R Latch ==
  S : Inport
  R : Inport  Port=2
  Constant1 : xbsIndex_r4/Constant  const=1  gui_display_data_type=Fixed-point  arith_type=Boolean  n_bits=1  bin_pt=0  preci_type=Single  exp_width=8  frac_width=24  explicit_period=off  period=1  equ=P=C  opselect=C  inp2=PCIN>>17  opr=+  inp1=P  carry=CIN  dbl_ovrd=off  block_type=constant
      settings: block_version=VER_STRING_GOES_HERE
  Register2 : xbsIndex_r4/Register  init=0  rst=on  en=on  dbl_ovrd=off  block_type=register
      settings: xl_area=[1 1 0 0 0 0 0]  block_version=VER_STRING_GOES_HERE
  Q : Outport
  wires:
  S/1 -> Register2/3
  Register2/1 -> Q/1
  R/1 -> Register2/2
  Constant1/1 -> Register2/1

== system ofdm_txrx_supermimo_coded/OFDM Rx MIMO/Packet_Timing_Control/S-R Latch1 ==
  S : Inport
  R : Inport  Port=2
  Constant1 : xbsIndex_r4/Constant  const=1  gui_display_data_type=Fixed-point  arith_type=Boolean  n_bits=1  bin_pt=0  preci_type=Single  exp_width=8  frac_width=24  explicit_period=off  period=1  equ=P=C  opselect=C  inp2=PCIN>>17  opr=+  inp1=P  carry=CIN  dbl_ovrd=off  block_type=constant
      settings: block_version=VER_STRING_GOES_HERE
  Register2 : xbsIndex_r4/Register  init=0  rst=on  en=on  dbl_ovrd=off  block_type=register
      settings: xl_area=[1 1 0 0 0 0 0]  block_version=VER_STRING_GOES_HERE
  Q : Outport
  wires:
  Constant1/1 -> Register2/1
  R/1 -> Register2/2
  Register2/1 -> Q/1
  S/1 -> Register2/3

== system ofdm_txrx_supermimo_coded/OFDM Tx MIMO/IFFT/Posedge2 ==
  D : Inport
  Delay : xbsIndex_r4/Delay  rst=off  infoeditControl=Selection of Reset will increase slice count due to use of real FFs and instead of SRLs  en=off  latency=1  dbl_ovrd=off  reg_retiming=off  block_type=delay
      settings: infoedit=Hardware notes: A delay line is a chain, each link of which is an SRL16 followed by a flip-flop. If register retiming is enabled, the delay line is a chain of flip-flops.  xl_area=[1 1 0 0 0 0 0]  block_version=VER_STRING_GOES_HERE
  Inverter : xbsIndex_r4/Inverter  en=off  latency=0  dbl_ovrd=off  block_type=inv
      settings: xl_area=[0 0 0 0 0 0 0]  block_version=VER_STRING_GOES_HERE
  Logical : xbsIndex_r4/Logical  logical_function=AND  inputs=2  en=off  latency=0  precision=Full  arith_type=Unsigned  n_bits=1  bin_pt=0  align_bp=on  dbl_ovrd=off  block_type=logical
      settings: xl_area=[1 0 0 1 0 0 0]  block_version=VER_STRING_GOES_HERE
  Q : Outport
  wires:
  Delay/1 -> Inverter/1
  Inverter/1 -> Logical/2
  D/1 -> Logical/1, Delay/1
  Logical/1 -> Q/1

== system ofdm_txrx_supermimo_coded/OFDM Tx MIMO/IFFT/SR Latch ==
  S : Inport
  R : Inport  Port=2
  Constant1 : xbsIndex_r4/Constant  const=1  gui_display_data_type=Fixed-point  arith_type=Boolean  n_bits=1  bin_pt=0  preci_type=Single  exp_width=8  frac_width=24  explicit_period=off  period=2  equ=P=C  opselect=C  inp2=PCIN>>17  opr=+  inp1=P  carry=CIN  dbl_ovrd=off  block_type=constant
      settings: block_version=VER_STRING_GOES_HERE
  Payload Running3 : xbsIndex_r4/Register  init=0  rst=on  en=on  dbl_ovrd=off  block_type=register
      settings: xl_area=[1 1 0 0 0 0 0]  block_version=VER_STRING_GOES_HERE
  Q : Outport
  wires:
  Constant1/1 -> Payload Running3/1
  R/1 -> Payload Running3/2
  S/1 -> Payload Running3/3
  Payload Running3/1 -> Q/1

== system ofdm_txrx_supermimo_coded/OFDM Tx MIMO/OutputBuffers/1 Shift Reg ==
  d : Inport
  rst : Inport  Port=2
  en : Inport  Port=3
  Payload Running1 : xbsIndex_r4/Register  init=0  rst=on  en=on  dbl_ovrd=off  block_type=register
      settings: xl_area=[1 1 0 0 0 0 0]  block_version=VER_STRING_GOES_HERE
  Q : Outport
  wires:
  en/1 -> Payload Running1/3
  rst/1 -> Payload Running1/2
  d/1 -> Payload Running1/1
  Payload Running1/1 -> Q/1

== system ofdm_txrx_supermimo_coded/OFDM Tx MIMO/OutputBuffers/1 Shift Reg2 ==
  d : Inport
  rst : Inport  Port=2
  en : Inport  Port=3
  Payload Running1 : xbsIndex_r4/Register  init=0  rst=on  en=on  dbl_ovrd=off  block_type=register
      settings: xl_area=[1 1 0 0 0 0 0]  block_version=VER_STRING_GOES_HERE
  Q : Outport
  wires:
  en/1 -> Payload Running1/3
  rst/1 -> Payload Running1/2
  d/1 -> Payload Running1/1
  Payload Running1/1 -> Q/1
